simulink model "ofdm_txrx_mimo_2x"  (Simulink 6.6)
settings: Solver=VariableStepDiscrete  StartTime=0.0  StopTime=20e3  RelTol=1e-3
PreLoadFcn:
  | ofdm_rx_mimo_init
InitFcn:
  | ofdm_rx_mimo_init
StartFcn:
  | ofdm_rx_mimo_init
values from neighbouring files (symbols this model uses):
  BER_RAM_init_size = 2048    [ofdm_tx_mimo_init.m]
  CPLength = 16    [ofdm_tx_mimo_init.m]
  CRCPolynomial = hex2dec('04c11db7')    [ofdm_tx_mimo_init.m]
  freqOffset_track_filtCoef_i = hex2dec('2500')/2^32    [ofdm_rx_mimo_init.m]
  freqOffset_track_filtCoef_p = hex2dec('d0000')/2^32    [ofdm_rx_mimo_init.m]
  hi = zeros(1,length(cci))    [ofdm_rx_mimo_init.m]
  hr = zeros(1,length(ccr))    [ofdm_rx_mimo_init.m]
  max_CP_length = 16    [ofdm_tx_mimo_init.m]
  max_num_baseRateSymbols = 31    [ofdm_tx_mimo_init.m]
  max_num_subcarriers = 64    [ofdm_tx_mimo_init.m]
  max_num_trainingSymbols = 15    [ofdm_tx_mimo_init.m]
  max_numBytes = 16384    [ofdm_tx_mimo_init.m]
  max_OFDM_symbols = 2047    [ofdm_tx_mimo_init.m]
  modConstellation_qam16 = .75*[1 3 -1 -3]./3    [ofdm_tx_mimo_init.m]
  modConstellation_qam256 = 0.9375*[3 1 5 7 11 9 13 15 -3 -1 -5 -7 -11 -9 -13 -15]./15    [ofdm_tx_mimo_init.m]
  modConstellation_qam64 = 0.875*[3 1 5 7 -3 -1 -5 -7]./7    [ofdm_tx_mimo_init.m]
  modConstellation_qpsk = [1 -1]./sqrt(2)    [ofdm_tx_mimo_init.m]
  numBaseRateSymbols = 2    [ofdm_tx_mimo_init.m]
  numSubcarriers = 64    [ofdm_tx_mimo_init.m]
  numTrainingSymbols = 2    [ofdm_tx_mimo_init.m]
  pilot1_indicies = 7 + ( (64-7) * 2^16)    (= 3735559)    [ofdm_tx_mimo_init.m]
  pilot2_indicies = 21 + ( (64-21) * 2^16)    (= 2818069)    [ofdm_tx_mimo_init.m]
  pilotValue_neg = hex2dec('8000') + (2^16 * 0)    [ofdm_tx_mimo_init.m]
  pilotValue_pos = hex2dec('7FFF') + (2^16 * 0)    [ofdm_tx_mimo_init.m]
  pkt_crossCorr_thresh = 0.7    [ofdm_rx_mimo_init.m]
  pkt_energy_thresh = 0    [ofdm_rx_mimo_init.m]
  pkt_numPayloadBytes = 1400+42-6    (= 1436)    [ofdm_tx_mimo_init.m]
  pktByteNums = numHeaderBytes +  				byteIndex_numPayloadBytes(1)*2^8 +  				byteIndex_numPayloadBytes(2)*2^16 +  				byteIndex_simpleDynModMasks * 2^24    [ofdm_rx_mimo_init.m]
  PktDet_Delay = 26 + Symbol_Timing_Offset*2^7    (= 1050)    [ofdm_rx_mimo_init.m]
  preamble = 6*[0 shortsyms_10 longsyms_2]    [ofdm_tx_mimo_init.m]
  preamble_I = real(preamble)    [ofdm_tx_mimo_init.m]
  preamble_Q = imag(preamble)    [ofdm_tx_mimo_init.m]
  RAM_init_size = max_numBytes    (= 16384)    [ofdm_tx_mimo_init.m]
  reg_Interrupt_PktBuf_Control = reg_InterruptControl +  	1 * 2^16 +  	0 * 2^24    (= 65656)    [ofdm_rx_mimo_init.m]
  rx_controlBits = 1 * 2  		 + 1 * 4  		 + 0 * 8  		 + 1 * 16 * rx_SISO_Mode  		 + 1 * 32  		 + 1 * 64  		 + 0 * 128  		 + 1 * 256  		 + 0 * 512  		 + 1 * 1024  		 + 0 * 2048  		 + 1 * 4096  		 + 1 * 8192  		 + 0 * 16384  		 + 1 * 65536  		 + 1 * 131072  		 + 0 * 262144  		 + 1 * 524288    [ofdm_rx_mimo_init.m]
  Rx_PhaseNoiseTrack_K = 0.6094    [ofdm_rx_mimo_init.m]
  rx_postEq_scaling = round(rxScaling*2^11) * (1 + 2^16)    [ofdm_rx_mimo_init.m]
  RxDataScrambling_Seq = [40 198 78 63 82 173 102 245 48 111 172 115 147 230 216 93 72 65 62 2 205 242 122 90 128 83 105 97 73 10 5 252]    [ofdm_tx_mimo_init.m]
  simOnly_numSamples = length(preamble)+( (numSubcarriers+CPLength)*(numTrainingSymbols + numBaseRateSymbols + numFullRateSymbols/2) )    [ofdm_tx_mimo_init.m]
  train = [train train]    [ofdm_tx_mimo_init.m]
  tx_controlBits = (antB_preambleShift * 2^4) + (2^0 * tx_SISO_Mode) + 4*0 + 8*1    (= 73)    [ofdm_tx_mimo_init.m]
  TxDataScrambling_Seq = [40 198 78 63 82 173 102 245 48 111 172 115 147 230 216 93 72 65 62 2 205 242 122 90 128 83 105 97 73 10 5 252]    [ofdm_tx_mimo_init.m]
  txReg_symbolCounts = (2^16 * numFullRateSymbols) + (2^8 *numBaseRateSymbols ) + numTrainingSymbols    [ofdm_tx_mimo_init.m]
  TxRx_FFTScaling = bin2dec('010111') + (bin2dec('000101') * 2^6)    [ofdm_tx_mimo_init.m]
undefined symbols (not defined in the file or in its folder): AntA, AntB, BaseRate, CRC_table_gen, gcbh, intfilt, pktTiming_count_payloadStart, pktTiming_symbol_length, xlBlockLoadCallback, xlGetSimulinkPeriod
libraries: xbsIndex_r4 x3973, dspsigops x4, commchan3 x2, commrflib2 x2, dspsrcs4 x1
(part 5 of 38)

== system ofdm_txrx_mimo_2x/OFDM Rx MIMO/Packet_Detection ==
  note: Only use one antenna for packet detection in this version
  note: Delay to match latencies of correlation &
      | energy calculation branches
  note: This block is used to detect the start of a packet. It correlates the energy in the received signal in order to
      | determine the packet start.  The signal will be detected in the preamble  (Usually during the third short symbol).
  note: Pkt Det goes high sometime
      | during the third short symbol
  note: Latency = 2
  note: Latency = 3
  note: Latency = 4
  note: Latency = 2
  note: Latency = 1
  AntA I : Inport
  AntA Q : Inport  Port=2
  AntB I : Inport  Port=3
  AntB Q : Inport  Port=4
  A*conj(B) 4xCLK : Subsystem
  Coarse Pkt Det : Scope  NumInputPorts=6
      settings: YMin=-0.03~-1~-1~-1~-1~-5  YMax=0.04~1~1~1~1~5  SaveName=ScopeData28
  Constant2 : Constant  Value=0
  Convert : xbsIndex_r4/Convert  arith_type="Signed  (2's comp)"  n_bits=18  bin_pt=11  quantization=Truncate  overflow=Flag as error  latency=0  dbl_ovrd=off  pipeline=off  block_type=convert
      settings: xl_area=[0 0 0 0 0 0 0]  block_version=VER_STRING_GOES_HERE
  Delay : xbsIndex_r4/Delay  en=off  latency=16  dbl_ovrd=off  reg_retiming=off  block_type=delay
      settings: infoedit=Hardware notes: A delay line is a chain, each link of which is an SRL16 followed by a flip-flop. If register retiming is enabled, the delay line is a chain of flip-flops.  xl_area=[7 14 0 14 0 0 0]  block_version=VER_STRING_GOES_HERE
  Delay1 : xbsIndex_r4/Delay  en=off  latency=16  dbl_ovrd=off  reg_retiming=off  block_type=delay
      settings: infoedit=Hardware notes: A delay line is a chain, each link of which is an SRL16 followed by a flip-flop. If register retiming is enabled, the delay line is a chain of flip-flops.  xl_area=[7 14 0 14 0 0 0]  block_version=VER_STRING_GOES_HERE
  Delay2 : xbsIndex_r4/Delay  Orientation=down  en=off  latency=3  dbl_ovrd=off  reg_retiming=off  block_type=delay
      settings: infoedit=Hardware notes: A delay line is a chain, each link of which is an SRL16 followed by a flip-flop. If register retiming is enabled, the delay line is a chain of flip-flops.  xl_area=[7 14 0 14 0 0 0]  block_version=VER_STRING_GOES_HERE
  Divide : Product  Inputs=*/  OutScaling=2^-10
  Down Sample : xbsIndex_r4/Down Sample  sample_ratio=2  sample_phase="Last Value of Frame  (most efficient)"  en=off  latency=1  dbl_ovrd=off  block_type=dsamp
      settings: block_version=VER_STRING_GOES_HERE
  Inverter1 : xbsIndex_r4/Inverter  en=off  latency=0  dbl_ovrd=off  block_type=inv
      settings: xl_area=[0 0 0 0 0 0 0]  block_version=VER_STRING_GOES_HERE
  Logical{,1..2,4} (4 alike) : xbsIndex_r4/Logical  logical_function=AND  inputs=2  en=off  latency=0  precision=Full  arith_type=Unsigned  n_bits=8  bin_pt=2  align_bp=on  dbl_ovrd=off  block_type=logical
      settings: xl_area=[1 0 0 1 0 0 0]  block_version=VER_STRING_GOES_HERE
  Logical3 : xbsIndex_r4/Logical  logical_function=OR  inputs=2  en=off  latency=0  precision=Full  arith_type=Unsigned  n_bits=8  bin_pt=2  align_bp=on  dbl_ovrd=off  block_type=logical
      settings: xl_area=[1 0 0 1 0 0 0]  block_version=VER_STRING_GOES_HERE
  Magnitude Squared : Subsystem  mask{prec=22, bp=14, multLatency=3, addLatency=1}
      mask prompts: prec "Precision"; bp "Binary Point"; multLatency "Multiplier Latency"; addLatency "Adder Latency"
  Magnitude Squared1 : Subsystem  mask{prec=17, bp=14, multLatency=2, addLatency=0}
      mask prompts: prec "Precision"; bp "Binary Point"; multLatency "Multiplier Latency"; addLatency "Adder Latency"
  Mult1 : xbsIndex_r4/Mult  precision=User Defined  arith_type=Unsigned  n_bits=18  bin_pt=10  quantization=Truncate  overflow=Flag as error  en=off  latency=2  dbl_ovrd=off  use_behavioral_HDL=off  use_embedded=on  opt=Speed  optimum_pipeline=off  pipeline=on  use_rpm=off  placement_style=Rectangular shape  block_type=mult
      settings: infoedit=Hardware notes: To use the internal pipeline stage of the dedicated multiplier you must select 'Pipeline for maximum performance'.  xl_area=[22 24 0 44 0 1 0]  block_version=VER_STRING_GOES_HERE
  Mult2 : xbsIndex_r4/Mult  precision=User Defined  arith_type=Unsigned  n_bits=22  bin_pt=14  quantization=Truncate  overflow=Flag as error  en=off  latency=2  dbl_ovrd=off  use_behavioral_HDL=off  use_embedded=on  opt=Speed  optimum_pipeline=off  pipeline=on  use_rpm=off  placement_style=Rectangular shape  block_type=mult
      settings: infoedit=Hardware notes: To use the internal pipeline stage of the dedicated multiplier you must select 'Pipeline for maximum performance'.  xl_area=[19 30 0 38 0 2 0]  block_version=VER_STRING_GOES_HERE
  Relational : xbsIndex_r4/Relational  mode=a>b  en=off  latency=1  dbl_ovrd=off  block_type=relational
      settings: xl_area=[11 1 0 22 0 0 0]  block_version=VER_STRING_GOES_HERE
  Relational1 : xbsIndex_r4/Relational  mode=a>b  en=off  latency=3  dbl_ovrd=off  block_type=relational
      settings: xl_area=[10 2 0 19 0 0 0]  block_version=VER_STRING_GOES_HERE
  Rx_ExtPktDet : xbsIndex_r4/Gateway In  arith_type=Boolean  n_bits=1  bin_pt=0  quantization="Round  (unbiased: +/- Inf)"  overflow=Saturate  period=4  dbl_ovrd=off  timing_constraint=None  locs_specified=off  LOCs={}  block_type=gatewayin
      settings: infoedit="Gateway in block.  Converts inputs of type Simulink integer, double and fixed point to  Xilinx fixed point type.<P><P>Hardware notes:  In hardware these blocks become top level input ports."  block_version=VER_STRING_GOES_HERE
  Slice1 : xbsIndex_r4/Slice  nbits=12  boolean_output=off  mode=Lower Bit Location + Width  bit1=0  base1=MSB of Input  bit0=8  base0=LSB of Input  dbl_ovrd=off  block_type=slice
      settings: infoedit="Extracts a given range of bits from each input sample and presents it at the output.  The output type is ordinarily unsigned with binary point at zero, but can be Boolean when the slice is one bit wide.<P><P>Hardware notes: In hardware this block costs nothing."  block_version=VER_STRING_GOES_HERE
  Slice25 : xbsIndex_r4/Slice  nbits=8  boolean_output=off  mode=Lower Bit Location + Width  bit1=0  base1=MSB of Input  bit0=0  base0=LSB of Input  dbl_ovrd=off  block_type=slice
      settings: infoedit="Extracts a given range of bits from each input sample and presents it at the output.  The output type is ordinarily unsigned with binary point at zero, but can be Boolean when the slice is one bit wide.<P><P>Hardware notes: In hardware this block costs nothing."  block_version=VER_STRING_GOES_HERE
  Sliding Window : Subsystem
  Sliding Window1 : Subsystem
  Sliding Window2 : Subsystem
  Terminator : Terminator
  Terminator1 : Terminator
  To_UFix12_5 : xbsIndex_r4/Reinterpret  force_arith_type=on  arith_type=Unsigned  force_bin_pt=on  bin_pt=5  block_type=cast
      settings: infoedit="Changes type of samples without altering their binary representation.<P><P>Hardware notes: In hardware this block costs nothing.<P><P>Example:  Suppose the input is 6 bits wide, signed, with 2 fractional bits, and the output is forced to unsigned with 0 fractional bits.  Then an input of -2.0 (1110.00 in binary 2's complement) becomes an output of 56 (111000 in binary)."  block_version=VER_STRING_GOES_HERE
  To_UFix8_7 : xbsIndex_r4/Reinterpret  force_arith_type=on  arith_type=Unsigned  force_bin_pt=on  bin_pt=7  block_type=cast
      settings: infoedit="Changes type of samples without altering their binary representation.<P><P>Hardware notes: In hardware this block costs nothing.<P><P>Example:  Suppose the input is 6 bits wide, signed, with 2 fractional bits, and the output is forced to unsigned with 0 fractional bits.  Then an input of -2.0 (1110.00 in binary 2's complement) becomes an output of 56 (111000 in binary)."  block_version=VER_STRING_GOES_HERE
  done{1,3..6} (5 alike) : xbsIndex_r4/Gateway Out  hdl_port=off  timing_constraint=None  locs_specified=off  LOCs={}  block_type=gatewayout
      settings: infoedit="Gateway out block.  Converts Xilinx fixed point inputs into ouputs of type Simulink integer, double, or fixed point.<P><P>Hardware notes:  In hardware these blocks become top level output ports or are discarded, depending on how they are configured."  xl_area=[0 0 0 0 1 0 0]  block_version=VER_STRING_GOES_HERE
  Pkt Det : Outport
  wires:
  Constant2/1 -> Rx_ExtPktDet/1
  Logical4/1 -> Logical3/2
  [regRx_enIntPktDet] -> Logical4/1
  [regRx_enExtPktDet] -> Logical2/2
  Logical3/1 -> Pkt Det/1
  Logical2/1 -> Logical3/1
  Rx_ExtPktDet/1 -> Logical2/1
  Divide/1 -> Coarse Pkt Det/6
  done6/1 -> Coarse Pkt Det/4
  done3/1 -> Divide/2, Coarse Pkt Det/3
  done5/1 -> Divide/1, Coarse Pkt Det/2
  done4/1 -> Coarse Pkt Det/1
  done1/1 -> Coarse Pkt Det/5
  Down Sample/1 -> Inverter1/1
  AntB Q/1 -> Terminator1/1
  AntB I/1 -> Terminator/1
  Delay2/1 -> done5/1, Relational/1
  Logical/1 -> done6/1, Logical1/1
  Logical1/1 -> Logical4/2, done1/1
  Inverter1/1 -> Logical1/2
  [rx_global_reset] -> Down Sample/1
  Relational1/1 -> Logical/2
  Convert/1 -> Mult1/2, Mult1/1
  Relational/1 -> Logical/1
  Magnitude Squared/1(Mag) -> Delay2/1
  Mult2/1 -> Relational/2
  Mult1/1 -> done3/1, Relational1/1, Mult2/1
  Sliding Window2/1(Out) -> Convert/1
  Magnitude Squared1/1(Mag) -> Sliding Window2/1(In)
  Sliding Window1/1(Out) -> Magnitude Squared/2(Q)
  Sliding Window/1(Out) -> Magnitude Squared/1(I)
  A*conj(B) 4xCLK/2(Im1) -> Sliding Window1/1(In)
  A*conj(B) 4xCLK/1(Re) -> Sliding Window/1(In)
  Delay1/1 -> Magnitude Squared1/2(Q), A*conj(B) 4xCLK/4(Im[B])
  Delay/1 -> Magnitude Squared1/1(I), A*conj(B) 4xCLK/2(Re[B])
  AntA I/1 -> done4/1, A*conj(B) 4xCLK/1(Re[A]), Delay/1
  AntA Q/1 -> A*conj(B) 4xCLK/3(Im[A]), Delay1/1
  Slice1/1 -> To_UFix12_5/1
  [Rx_IntPktDet_Thresholds] -> Slice25/1, Slice1/1
  Slice25/1 -> To_UFix8_7/1
  To_UFix8_7/1 -> Mult2/2
  To_UFix12_5/1 -> Relational1/2

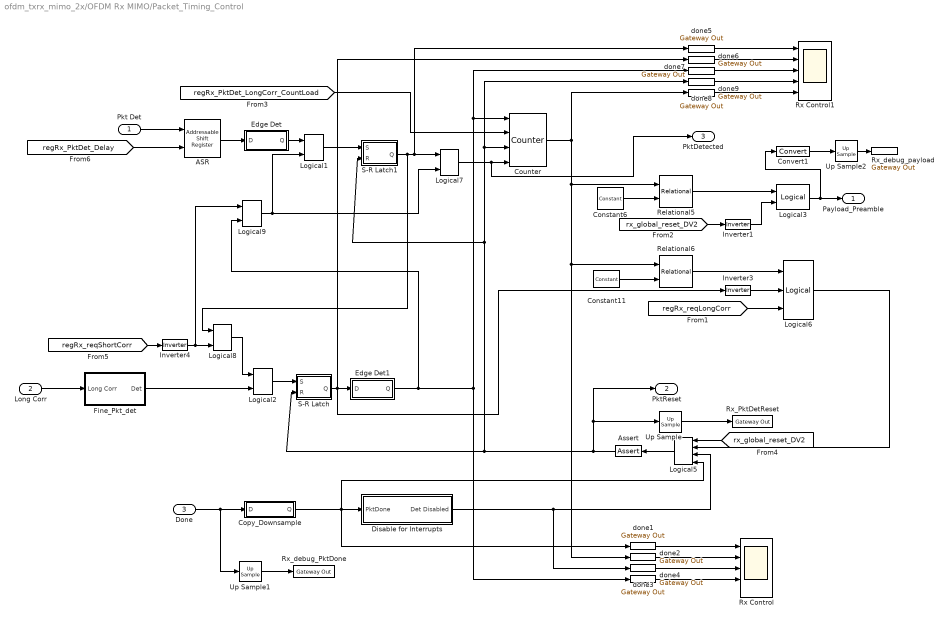
== system ofdm_txrx_mimo_2x/OFDM Rx MIMO/Packet_Timing_Control ==
  Pkt Det : Inport
  Long Corr : Inport  Port=2
  Done : Inport  Port=3
  ASR : xbsIndex_r4/Addressable Shift Register  infer_latency=on  depth=2  initVector=[0]  en=off  dbl_ovrd=off  use_behavioral_HDL=off  use_rpm=off  block_type=addrsr
      settings: infoedit="Delay of  configurable length.  Any element  in the delay     line   can    be   addressed   and   driven    onto   the   output     port.<P><P>Hardware  notes: Implemented  using SRL16s.     If Virtex-4, Virtex-II or Spartan-3 devices are used, multiple SRLC16s are cascaded together."  xl_area=[13 0 0 25 0 0 0]  block_version=VER_STRING_GOES_HERE
  Assert : xbsIndex_r4/Assert  Orientation=left  assert_type=on  type_source=Explicitly  arith_type=Boolean  n_bits=16  bin_pt=14  assert_rate=on  rate_source=Explicitly  period=4  output_port=on  block_type=assert
      settings: infoedit=Asserts a user-defined sample rate and/or type on a signal.<P><P>Hardware notes: In hardware this block costs nothing.  block_version=VER_STRING_GOES_HERE
  Constant11 : xbsIndex_r4/Constant  arith_type=Unsigned  const=pktTiming_count_payloadStart-2  n_bits=ceil(log2(pktTiming_count_payloadStart))  bin_pt=0  explicit_period=off  period=1  equ=P=C  opselect=C  inp2=PCIN>>17  opr=+  inp1=P  carry=CIN  dbl_ovrd=off  block_type=constant
      settings: block_version=VER_STRING_GOES_HERE
  Constant6 : xbsIndex_r4/Constant  arith_type=Unsigned  const=pktTiming_count_payloadStart  n_bits=ceil(log2(pktTiming_count_payloadStart))+1  bin_pt=0  explicit_period=off  period=1  equ=P=C  opselect=C  inp2=PCIN>>17  opr=+  inp1=P  carry=CIN  dbl_ovrd=off  block_type=constant
      settings: block_version=VER_STRING_GOES_HERE
  Convert1 : xbsIndex_r4/Convert  arith_type=Unsigned  n_bits=1  bin_pt=0  quantization=Truncate  overflow=Wrap  latency=0  dbl_ovrd=off  pipeline=off  block_type=convert
      settings: xl_area=[0, 0, 0, 0, 0, 0, 0]  block_version=VER_STRING_GOES_HERE
  Copy_Downsample : Subsystem
  Counter : xbsIndex_r4/Counter  cnt_type=Free Running  cnt_to=Inf  operation=Up  start_count=0  cnt_by_val=1  arith_type=Unsigned  n_bits=pktTiming_controlCounter_bits  bin_pt=0  load_pin=on  rst=on  en=on  explicit_period=off  period=1  dbl_ovrd=off  use_behavioral_HDL=off  use_rpm=on  implementation=Fabric  block_type=counter
      settings: xl_area=[6 11 0 12 0 0 0]  block_version=VER_STRING_GOES_HERE
  Disable for Interrupts : Subsystem
  Edge Det : Subsystem
  Edge Det1 : Subsystem
  Fine_Pkt_det : Subsystem
  Inverter1 : xbsIndex_r4/Inverter  en=off  latency=0  dbl_ovrd=off  block_type=inv
      settings: xl_area=[0 0 0 0 0 0 0]  block_version=VER_STRING_GOES_HERE
  Inverter3 : xbsIndex_r4/Inverter  en=off  latency=0  dbl_ovrd=off  block_type=inv
      settings: xl_area=[0 0 0 0 0 0 0]  block_version=VER_STRING_GOES_HERE
  Inverter4 : xbsIndex_r4/Inverter  en=off  latency=0  dbl_ovrd=off  block_type=inv
      settings: xl_area=[0 0 0 0 0 0 0]  block_version=VER_STRING_GOES_HERE
  Logical1 : xbsIndex_r4/Logical  logical_function=OR  inputs=2  en=off  latency=0  precision=Full  arith_type=Unsigned  n_bits=8  bin_pt=2  align_bp=on  dbl_ovrd=off  block_type=logical
      settings: xl_area=[1 0 0 1 0 0 0]  block_version=VER_STRING_GOES_HERE
  Logical2 : xbsIndex_r4/Logical  logical_function=AND  inputs=2  en=off  latency=0  precision=Full  arith_type=Unsigned  n_bits=8  bin_pt=2  align_bp=on  dbl_ovrd=off  block_type=logical
      settings: xl_area=[1 0 0 1 0 0 0]  block_version=VER_STRING_GOES_HERE
  Logical3 : xbsIndex_r4/Logical  logical_function=AND  inputs=2  en=off  latency=0  precision=Full  arith_type=Unsigned  n_bits=8  bin_pt=2  align_bp=on  dbl_ovrd=off  block_type=logical
      settings: xl_area=[1 0 0 1 0 0 0]  block_version=VER_STRING_GOES_HERE
  Logical5 : xbsIndex_r4/Logical  Orientation=left  logical_function=OR  inputs=4  en=off  latency=0  precision=Full  arith_type=Unsigned  n_bits=8  bin_pt=2  align_bp=on  dbl_ovrd=off  block_type=logical
      settings: xl_area=[1 0 0 1 0 0 0]  block_version=VER_STRING_GOES_HERE
  Logical6 : xbsIndex_r4/Logical  logical_function=AND  inputs=3  en=off  latency=0  precision=Full  arith_type=Unsigned  n_bits=8  bin_pt=2  align_bp=on  dbl_ovrd=off  block_type=logical
      settings: xl_area=[1 0 0 1 0 0 0]  block_version=VER_STRING_GOES_HERE
  Logical7 : xbsIndex_r4/Logical  logical_function=OR  inputs=2  en=off  latency=0  precision=Full  arith_type=Unsigned  n_bits=8  bin_pt=2  align_bp=on  dbl_ovrd=off  block_type=logical
      settings: xl_area=[1 0 0 1 0 0 0]  block_version=VER_STRING_GOES_HERE
  Logical8 : xbsIndex_r4/Logical  logical_function=OR  inputs=2  en=off  latency=0  precision=Full  arith_type=Unsigned  n_bits=8  bin_pt=2  align_bp=on  dbl_ovrd=off  block_type=logical
      settings: xl_area=[1 0 0 1 0 0 0]  block_version=VER_STRING_GOES_HERE
  Logical9 : xbsIndex_r4/Logical  logical_function=AND  inputs=2  en=off  latency=0  precision=Full  arith_type=Unsigned  n_bits=8  bin_pt=2  align_bp=on  dbl_ovrd=off  block_type=logical
      settings: xl_area=[1 0 0 1 0 0 0]  block_version=VER_STRING_GOES_HERE
  Relational5 : xbsIndex_r4/Relational  mode=a>b  en=off  latency=0  dbl_ovrd=off  block_type=relational
      settings: xl_area=[6 0 0 11 0 0 0]  block_version=VER_STRING_GOES_HERE
  Relational6 : xbsIndex_r4/Relational  mode=a>b  en=off  latency=0  dbl_ovrd=off  block_type=relational
      settings: xl_area=[6 0 0 11 0 0 0]  block_version=VER_STRING_GOES_HERE
  Rx Control : Scope  NumInputPorts=5
      settings: TimeRange=2500  YMin=0.95~885~-1~-1~-1  YMax=1.05~935~1~1~1  SaveName=ScopeData13
  Rx Control1 : Scope  NumInputPorts=5
      settings: TimeRange=2500  YMin=1~1~-1~-1~605  YMax=1~1~1~1~665  SaveName=ScopeData47
  Rx_PktDetReset : xbsIndex_r4/Gateway Out  hdl_port=on  timing_constraint=None  locs_specified=off  LOCs={}  block_type=gatewayout
      settings: infoedit="Gateway out block.  Converts Xilinx fixed point inputs into ouputs of type Simulink integer, double, or fixed point.<P><P>Hardware notes:  In hardware these blocks become top level output ports or are discarded, depending on how they are configured."  xl_area=[0, 0, 0, 0, 0, 0, 0]  block_version=VER_STRING_GOES_HERE
  Rx_debug_PktDone : xbsIndex_r4/Gateway Out  hdl_port=on  timing_constraint=None  locs_specified=off  LOCs={}  block_type=gatewayout
      settings: infoedit="Gateway out block.  Converts Xilinx fixed point inputs into ouputs of type Simulink integer, double, or fixed point.<P><P>Hardware notes:  In hardware these blocks become top level output ports or are discarded, depending on how they are configured."  xl_area=[0, 0, 0, 0, 0, 0, 0]  block_version=VER_STRING_GOES_HERE
  Rx_debug_payload : xbsIndex_r4/Gateway Out  hdl_port=on  timing_constraint=None  locs_specified=off  LOCs={}  block_type=gatewayout
      settings: infoedit="Gateway out block.  Converts Xilinx fixed point inputs into ouputs of type Simulink integer, double, or fixed point.<P><P>Hardware notes:  In hardware these blocks become top level output ports or are discarded, depending on how they are configured."  xl_area=[0 0 0 0 1 0 0]  block_version=VER_STRING_GOES_HERE
  S-R Latch : Subsystem
  S-R Latch1 : Subsystem
  Up Sample : xbsIndex_r4/Up Sample  sample_ratio=4  copy_samples=on  dbl_ovrd=off  block_type=usamp
      settings: infoedit="Up samples input data.  Inserted values  can be zeros or copies of the most recent input sample.<P><P>Hardware notes: No hardware is needed if inserted values are copies of the input sample; otherwise, a mux and single bit flip-flop are used."  block_version=8.2.02
  Up Sample1 : xbsIndex_r4/Up Sample  sample_ratio=2  copy_samples=on  dbl_ovrd=off  block_type=usamp
      settings: infoedit="Up samples input data.  Inserted values  can be zeros or copies of the most recent input sample.<P><P>Hardware notes: No hardware is needed if inserted values are copies of the input sample; otherwise, a mux and single bit flip-flop are used."  block_version=8.2.02
  Up Sample2 : xbsIndex_r4/Up Sample  sample_ratio=4  copy_samples=on  dbl_ovrd=off  block_type=usamp
      settings: infoedit="Up samples input data.  Inserted values  can be zeros or copies of the most recent input sample.<P><P>Hardware notes: No hardware is needed if inserted values are copies of the input sample; otherwise, a mux and single bit flip-flop are used."  block_version=8.2.02
  done{1..9} (9 alike) : xbsIndex_r4/Gateway Out  hdl_port=off  timing_constraint=None  locs_specified=off  LOCs={}  block_type=gatewayout
      settings: infoedit="Gateway out block.  Converts Xilinx fixed point inputs into ouputs of type Simulink integer, double, or fixed point.<P><P>Hardware notes:  In hardware these blocks become top level output ports or are discarded, depending on how they are configured."  xl_area=[0 0 0 0 1 0 0]  block_version=VER_STRING_GOES_HERE
  Payload_Preamble : Outport
  PktReset : Outport  Port=2
  PktDetected : Outport  Port=3
  wires:
  Convert1/1 -> Up Sample2/1
  done9/1 -> Rx Control1/5
  done8/1 -> Rx Control1/4
  done7/1 -> Rx Control1/3
  done6/1 -> Rx Control1/2
  done5/1 -> Rx Control1/1
  Copy_Downsample/1(Q) -> done1/1, Logical5/4, Disable for Interrupts/1(PktDone)
  Assert/1 -> S-R Latch1/2(R), done8/1, Counter/3, S-R Latch/2(R), PktReset/1, Up Sample/1
  Disable for Interrupts/1(Det Disabled) -> done3/1, Logical5/3
  Done/1 -> Copy_Downsample/1(D), Up Sample1/1
  S-R Latch1/1(Q) -> done5/1, Logical7/1, Logical8/1
  Logical1/1 -> S-R Latch1/1(S)
  S-R Latch/1(Q) -> done6/1, Inverter3/1, Edge Det1/1(D)
  Edge Det1/1(Q) -> done7/1, Counter/1, done4/1, Logical9/2
  Logical9/1 -> Logical7/2, Logical1/2
  [regRx_PktDet_LongCorr_CountLoad] -> Counter/2
  Logical8/1 -> Logical2/1
  Inverter4/1 -> Logical9/1, Logical8/2
  [regRx_reqShortCorr] -> Inverter4/1
  Logical2/1 -> S-R Latch/1(S)
  Long Corr/1 -> Fine_Pkt_det/1(Long Corr)
  done4/1 -> Rx Control/4
  done3/1 -> Rx Control/3
  done2/1 -> Rx Control/2
  done1/1 -> Rx Control/1
  Logical7/1 -> Counter/4, PktDetected/1
  Logical6/1 -> Logical5/2
  [regRx_reqLongCorr] -> Logical6/3
  Inverter3/1 -> Logical6/2
  Relational6/1 -> Logical6/1
  Constant11/1 -> Relational6/2
  [rx_global_reset_DV2] -> Logical5/1
  Logical5/1 -> Assert/1
  Logical3/1 -> Convert1/1, Payload_Preamble/1
  Relational5/1 -> Logical3/1
  Inverter1/1 -> Logical3/2
  [rx_global_reset_DV2] -> Inverter1/1
  [regRx_PktDet_Delay] -> ASR/2
  ASR/1 -> Edge Det/1(D)
  Pkt Det/1 -> ASR/1
  Constant6/1 -> Relational5/2
  Edge Det/1(Q) -> Logical1/1
  Counter/1 -> done2/1, Relational6/1, Relational5/1, done9/1
  Fine_Pkt_det/1(Det) -> Logical2/2
  Up Sample/1 -> Rx_PktDetReset/1
  Up Sample1/1 -> Rx_debug_PktDone/1
  Up Sample2/1 -> Rx_debug_payload/1

== system ofdm_txrx_mimo_2x/OFDM Rx MIMO/Rx Reset Logic ==
  Convert : xbsIndex_r4/Convert  arith_type=Boolean  n_bits=8  bin_pt=6  quantization=Truncate  overflow=Wrap  latency=0  dbl_ovrd=off  pipeline=off  block_type=convert
      settings: xl_area=[0, 0, 0, 0, 0, 0, 0]  block_version=VER_STRING_GOES_HERE
  Down Sample6 : xbsIndex_r4/Down Sample  sample_ratio=2  sample_phase="Last Value of Frame  (most efficient)"  en=off  latency=1  dbl_ovrd=off  block_type=dsamp
      settings: block_version=VER_STRING_GOES_HERE
  Logical1 : xbsIndex_r4/Logical  logical_function=OR  inputs=2  en=off  latency=0  precision=Full  arith_type=Unsigned  n_bits=8  bin_pt=2  align_bp=on  dbl_ovrd=off  block_type=logical
      settings: xl_area=[1 0 0 1 0 0 0]  block_version=VER_STRING_GOES_HERE
  RXreset : Constant  Value=0
  Rx_Reset : xbsIndex_r4/Gateway In  arith_type=Boolean  n_bits=6  bin_pt=0  quantization="Round  (unbiased: +/- Inf)"  overflow=Saturate  period=4  dbl_ovrd=off  timing_constraint=None  locs_specified=off  LOCs={}  block_type=gatewayin
      settings: infoedit="Gateway in block.  Converts inputs of type Simulink integer, double and fixed point to  Xilinx fixed point type.<P><P>Hardware notes:  In hardware these blocks become top level input ports."  xl_area=[0 0 0 0 1 0 0]  block_version=VER_STRING_GOES_HERE
  tag scope: rx_global_reset=global, rx_global_reset_DV2=global
  wires:
  RXreset/1 -> Rx_Reset/1
  [regRx_GlobalReset] -> Logical1/1
  Logical1/1 -> [rx_global_reset], Down Sample6/1
  Rx_Reset/1 -> Convert/1
  Convert/1 -> Logical1/2
  Down Sample6/1 -> [rx_global_reset_DV2]

== system ofdm_txrx_mimo_2x/OFDM Tx MIMO/Filter & DACs ==
  A_I : Inport
  A_Q : Inport  Port=2
  B_I : Inport  Port=3
  B_Q : Inport  Port=4
  Convert : xbsIndex_r4/Convert  arith_type=Boolean  n_bits=16  bin_pt=14  quantization=Truncate  overflow=Wrap  latency=0  dbl_ovrd=off  pipeline=off  block_type=convert
      settings: block_version=8.2.02
  Down Sample : xbsIndex_r4/Down Sample  sample_ratio=2  sample_phase="Last Value of Frame  (most efficient)"  en=off  latency=1  dbl_ovrd=off  block_type=dsamp
      settings: block_version=10.1
  Logical : xbsIndex_r4/Logical  logical_function=AND  inputs=2  en=off  latency=0  precision=Full  arith_type=Unsigned  n_bits=16  bin_pt=0  align_bp=on  dbl_ovrd=off  block_type=logical
      settings: block_version=8.2.02
  Mux : xbsIndex_r4/Mux  inputs=2  en=off  latency=1  precision=Full  arith_type=Unsigned  n_bits=16  bin_pt=14  quantization=Truncate  overflow=Wrap  dbl_ovrd=off  block_type=mux
      settings: block_version=8.2.02
  Mux1 : xbsIndex_r4/Mux  inputs=2  en=off  latency=1  precision=Full  arith_type=Unsigned  n_bits=16  bin_pt=14  quantization=Truncate  overflow=Wrap  dbl_ovrd=off  block_type=mux
      settings: block_version=8.2.02
  Polyphase 2x Interpolator1 : Subsystem  mask{h=[0 intfilt(2,4,0.8)] .* 0.99, polyphaseBranches=2, data_samplePeriod=4, coeff_prec=14, coeff_bp=13, multLatency=1, mult_prec=18, mult_bp=17, add_prec=16, add_bp=15}
      mask prompts: h "Filter Coefficients"; polyphaseBranches "Interpolation Rate"; data_samplePeriod "Input Sample Period"; coeff_prec "Coefficients - Num Bits"; coeff_bp "Coefficients - Binary Point"; multLatency "Multiplier Latency"; mult_prec "Multiplier - Num Bits"; mult_bp "Multiplier - Binary Point"; add_prec "Final Adder - Num Bits"; add_bp "Final Adder - Binary Point"
      mask help:
      | This block implements a interpolating polyphase FIR filter. In this version,
      | the interpolation rate must be 8, and the filter must be of length 64.
      mask init:
      | subFilterLength = 2;
  Polyphase 2x Interpolator2 : Subsystem  mask{h=[0 intfilt(2,4,0.8)] .* 0.99, polyphaseBranches=2, data_samplePeriod=4, coeff_prec=14, coeff_bp=13, multLatency=1, mult_prec=18, mult_bp=17, add_prec=16, add_bp=15}
      mask prompts: h "Filter Coefficients"; polyphaseBranches "Interpolation Rate"; data_samplePeriod "Input Sample Period"; coeff_prec "Coefficients - Num Bits"; coeff_bp "Coefficients - Binary Point"; multLatency "Multiplier Latency"; mult_prec "Multiplier - Num Bits"; mult_bp "Multiplier - Binary Point"; add_prec "Final Adder - Num Bits"; add_bp "Final Adder - Binary Point"
      mask help:
      | This block implements a interpolating polyphase FIR filter. In this version,
      | the interpolation rate must be 8, and the filter must be of length 64.
      mask init:
      | subFilterLength = 2;
  Polyphase 2x Interpolator3 : Subsystem  mask{h=[0 intfilt(2,4,0.8)] .* 0.99, polyphaseBranches=2, data_samplePeriod=4, coeff_prec=14, coeff_bp=13, multLatency=1, mult_prec=18, mult_bp=17, add_prec=16, add_bp=15}
      mask prompts: h "Filter Coefficients"; polyphaseBranches "Interpolation Rate"; data_samplePeriod "Input Sample Period"; coeff_prec "Coefficients - Num Bits"; coeff_bp "Coefficients - Binary Point"; multLatency "Multiplier Latency"; mult_prec "Multiplier - Num Bits"; mult_bp "Multiplier - Binary Point"; add_prec "Final Adder - Num Bits"; add_bp "Final Adder - Binary Point"
      mask help:
      | This block implements a interpolating polyphase FIR filter. In this version,
      | the interpolation rate must be 8, and the filter must be of length 64.
      mask init:
      | subFilterLength = 2;
  Polyphase 2x Interpolator4 : Subsystem  mask{h=[0 intfilt(2,4,0.8)] .* 0.99, polyphaseBranches=2, data_samplePeriod=4, coeff_prec=14, coeff_bp=13, multLatency=1, mult_prec=18, mult_bp=17, add_prec=16, add_bp=15}
      mask prompts: h "Filter Coefficients"; polyphaseBranches "Interpolation Rate"; data_samplePeriod "Input Sample Period"; coeff_prec "Coefficients - Num Bits"; coeff_bp "Coefficients - Binary Point"; multLatency "Multiplier Latency"; mult_prec "Multiplier - Num Bits"; mult_bp "Multiplier - Binary Point"; add_prec "Final Adder - Num Bits"; add_bp "Final Adder - Binary Point"
      mask help:
      | This block implements a interpolating polyphase FIR filter. In this version,
      | the interpolation rate must be 8, and the filter must be of length 64.
      mask init:
      | subFilterLength = 2;
  Register : xbsIndex_r4/Register  init=0  rst=off  en=off  dbl_ovrd=off  block_type=register
  Register1 : xbsIndex_r4/Register  init=0  rst=off  en=off  dbl_ovrd=off  block_type=register
  To Fix16_{1..3,15} (4 alike) : xbsIndex_r4/Convert  arith_type="Signed  (2's comp)"  n_bits=16  bin_pt=15  quantization=Truncate  overflow=Wrap  latency=0  dbl_ovrd=off  pipeline=off  block_type=convert
      settings: block_version=10.1
  Tx_AntA_DAC_I : xbsIndex_r4/Gateway Out  hdl_port=on  timing_constraint=None  locs_specified=off  LOCs={}  block_type=gatewayout
      settings: infoedit="Gateway out block.  Converts Xilinx fixed point inputs into ouputs of type Simulink integer, double, or fixed point.<P><P>Hardware notes:  In hardware these blocks become top level output ports or are discarded, depending on how they are configured."  block_version=10.1.3
  Tx_AntA_DAC_Q : xbsIndex_r4/Gateway Out  hdl_port=on  timing_constraint=None  locs_specified=off  LOCs={}  block_type=gatewayout
      settings: infoedit="Gateway out block.  Converts Xilinx fixed point inputs into ouputs of type Simulink integer, double, or fixed point.<P><P>Hardware notes:  In hardware these blocks become top level output ports or are discarded, depending on how they are configured."  block_version=10.1.3
  Tx_AntB_DAC_I : xbsIndex_r4/Gateway Out  hdl_port=on  timing_constraint=None  locs_specified=off  LOCs={}  block_type=gatewayout
      settings: infoedit="Gateway out block.  Converts Xilinx fixed point inputs into ouputs of type Simulink integer, double, or fixed point.<P><P>Hardware notes:  In hardware these blocks become top level output ports or are discarded, depending on how they are configured."  block_version=10.1.3
  Tx_AntB_DAC_Q : xbsIndex_r4/Gateway Out  hdl_port=on  timing_constraint=None  locs_specified=off  LOCs={}  block_type=gatewayout
      settings: infoedit="Gateway out block.  Converts Xilinx fixed point inputs into ouputs of type Simulink integer, double, or fixed point.<P><P>Hardware notes:  In hardware these blocks become top level output ports or are discarded, depending on how they are configured."  block_version=10.1.3
  " A_I" : Outport
  " A_Q" : Outport  Port=2
  " B_I" : Outport  Port=3
  " B_Q" : Outport  Port=4
  wires:
  Polyphase 2x Interpolator3/1(Y) -> Mux/3, Register/1
  Tx_AntA_DAC_I/1 -> " A_I"/1
  Polyphase 2x Interpolator1/1(Y) -> Mux1/3, Register1/1
  Tx_AntA_DAC_Q/1 -> " A_Q"/1
  Polyphase 2x Interpolator2/1(Y) -> Mux/2
  Tx_AntB_DAC_I/1 -> " B_I"/1
  Polyphase 2x Interpolator4/1(Y) -> Mux1/2
  Tx_AntB_DAC_Q/1 -> " B_Q"/1
  Mux/1 -> To Fix16_2/1
  Mux1/1 -> To Fix16_3/1
  Logical/1 -> Down Sample/1
  Register/1 -> To Fix16_15/1
  Register1/1 -> To Fix16_1/1
  [TxReg_SISO_Mode] -> Logical/1
  [TxReg_SISO_AntB] -> Convert/1
  Convert/1 -> Logical/2
  Down Sample/1 -> Mux/1, Mux1/1
  To Fix16_15/1 -> Tx_AntA_DAC_I/1
  To Fix16_1/1 -> Tx_AntA_DAC_Q/1
  To Fix16_2/1 -> Tx_AntB_DAC_I/1
  To Fix16_3/1 -> Tx_AntB_DAC_Q/1
  A_I/1 -> Polyphase 2x Interpolator3/1(X)
  A_Q/1 -> Polyphase 2x Interpolator1/1(X)
  B_I/1 -> Polyphase 2x Interpolator2/1(X)
  B_Q/1 -> Polyphase 2x Interpolator4/1(X)

== system ofdm_txrx_mimo_2x/OFDM Tx MIMO/IFFT ==
  xn_I : Inport
  xn_Q : Inport  Port=2
  Start : Inport  Port=3
  Constant3 : xbsIndex_r4/Constant  arith_type=Boolean  const=0  n_bits=1  bin_pt=0  explicit_period=off  period=1  equ=P=C  opselect=C  inp2=PCIN>>17  opr=+  inp1=P  carry=CIN  dbl_ovrd=off  block_type=constant
      settings: block_version=VER_STRING_GOES_HERE
  Constant4 : xbsIndex_r4/Constant  arith_type=Boolean  const=1  n_bits=1  bin_pt=0  explicit_period=off  period=1  equ=P=C  opselect=C  inp2=PCIN>>17  opr=+  inp1=P  carry=CIN  dbl_ovrd=off  block_type=constant
      settings: block_version=VER_STRING_GOES_HERE
  Constant6 : xbsIndex_r4/Constant  arith_type=Boolean  const=1  n_bits=1  bin_pt=0  explicit_period=off  period=1  equ=P=C  opselect=C  inp2=PCIN>>17  opr=+  inp1=P  carry=CIN  dbl_ovrd=off  block_type=constant
      settings: block_version=VER_STRING_GOES_HERE
  FFTx : xbsIndex_r4/FFT v3_1   arch=Pipelined Streaming I/O  npts=64  scaling=Scaled  rounding=Convergent Rounding  tf_width=16  en_n=off  has_ovflo=on  rst=on  en=off  op_order=Natural Order  dbl_ovrd=off  explicit_period=off  period=4  use_dsp48=off  twiddle_mem_type=Block RAM  data_mem_type=Block RAM  num_bram_stages=3  block_type=fftx
      settings: infoedit=Devices Supported: Virtex-4, Virtex-II, Virtex-IIPro, Spartan-3 and Spartan-3E  xl_area=[0, 0, 0, 0, 0, 0, 0]  block_version=VER_STRING_GOES_HERE
  IFFT Input : Scope  NumInputPorts=4
      settings: TimeRange=4000  YMin=-0.8~-0.8~-1~0  YMax=1~0.8~1~65  SaveName=ScopeData16
  IFFT Output : Scope  NumInputPorts=6
      settings: TimeRange=4000  YMin=-0.6~-0.6~0~0~0~-1  YMax=0.2~0.2~70~1~1~1  SaveName=ScopeData17
  IFFT Output1 : Scope  NumInputPorts=6
      settings: TimeRange=4000  YMin=-0.6~-0.6~0~0~0~-1  YMax=0.2~0.2~70~1~1~1  SaveName=ScopeData69
  done{1..10} (10 alike) : xbsIndex_r4/Gateway Out  hdl_port=off  timing_constraint=None  locs_specified=off  LOCs={}  block_type=gatewayout
      settings: infoedit="Gateway out block.  Converts Xilinx fixed point inputs into ouputs of type Simulink integer, double, or fixed point.<P><P>Hardware notes:  In hardware these blocks become top level output ports or are discarded, depending on how they are configured."  xl_area=[0 0 0 0 1 0 0]  block_version=VER_STRING_GOES_HERE
  xn_Index : Outport
  Vout : Outport  Port=2
  xk_I : Outport  Port=3
  xk_Q : Outport  Port=4
  xk_Index : Outport  Port=5
  wires:
  Constant3/1 -> FFTx/4
  Constant4/1 -> FFTx/5
  Constant6/1 -> FFTx/7
  [TxReset] -> FFTx/8
  xn_I/1 -> FFTx/1, done4/1
  FFTx/1 -> xk_I/1, done8/1
  FFTx/2 -> xk_Q/1, done9/1
  xn_Q/1 -> FFTx/2, done5/1
  FFTx/3 -> xn_Index/1, done6/1, IFFT Output1/2
  Start/1 -> FFTx/3, done3/1, IFFT Output1/1
  FFTx/4 -> xk_Index/1, done7/1, IFFT Output1/3
  FFTx/7 -> Vout/1, done10/1, IFFT Output1/4
  done4/1 -> IFFT Input/1
  done5/1 -> IFFT Input/2
  done3/1 -> IFFT Input/3
  done6/1 -> IFFT Input/4
  done8/1 -> IFFT Output/1
  done9/1 -> IFFT Output/2
  done7/1 -> IFFT Output/3
  done10/1 -> IFFT Output/4
  done2/1 -> IFFT Output/5
  FFTx/9 -> done2/1, IFFT Output1/5
  done1/1 -> IFFT Output/6
  FFTx/10 -> done1/1
  [Tx_FFTScaling] -> FFTx/6

== system ofdm_txrx_mimo_2x/OFDM Tx MIMO/OutputBuffers ==
  IFFT Vout : Inport
  I : Inport  Port=2
  Q : Inport  Port=3
  Xk_index : Inport  Port=4
  1 Shift Reg : Subsystem
  Addr Gen : Subsystem
  Constant2 : xbsIndex_r4/Constant  arith_type=Boolean  const=0  n_bits=0  bin_pt=0  explicit_period=off  period=1  equ=P=C  opselect=C  inp2=PCIN>>17  opr=+  inp1=P  carry=CIN  dbl_ovrd=off  block_type=constant
      settings: block_version=VER_STRING_GOES_HERE
  Convert1 : xbsIndex_r4/Convert  arith_type="Signed  (2's comp)"  n_bits=14  bin_pt=13  quantization=Truncate  overflow=Flag as error  latency=0  dbl_ovrd=off  pipeline=off  block_type=convert
      settings: xl_area=[0 0 0 0 0 0 0]  block_version=VER_STRING_GOES_HERE
  Convert2 : xbsIndex_r4/Convert  arith_type="Signed  (2's comp)"  n_bits=14  bin_pt=13  quantization=Truncate  overflow=Flag as error  latency=0  dbl_ovrd=off  pipeline=off  block_type=convert
      settings: xl_area=[0 0 0 0 0 0 0]  block_version=VER_STRING_GOES_HERE
  Delay : xbsIndex_r4/Delay  en=off  latency=1  dbl_ovrd=off  reg_retiming=off  block_type=delay
      settings: infoedit=Hardware notes: A delay line is a chain, each link of which is an SRL16 followed by a flip-flop. If register retiming is enabled, the delay line is a chain of flip-flops.  block_version=VER_STRING_GOES_HERE
  Logical : xbsIndex_r4/Logical  logical_function=AND  inputs=2  en=off  latency=0  precision=Full  arith_type=Unsigned  n_bits=16  bin_pt=0  align_bp=on  dbl_ovrd=off  block_type=logical
      settings: block_version=VER_STRING_GOES_HERE
  Logical1 : xbsIndex_r4/Logical  logical_function=AND  inputs=2  en=off  latency=0  precision=Full  arith_type=Unsigned  n_bits=16  bin_pt=0  align_bp=on  dbl_ovrd=off  block_type=logical
      settings: block_version=VER_STRING_GOES_HERE
  Logical2 : xbsIndex_r4/Logical  logical_function=OR  inputs=2  en=off  latency=0  precision=Full  arith_type=Unsigned  n_bits=16  bin_pt=0  align_bp=on  dbl_ovrd=off  block_type=logical
      settings: block_version=VER_STRING_GOES_HERE
  Logical3 : xbsIndex_r4/Logical  logical_function=AND  inputs=2  en=off  latency=0  precision=Full  arith_type=Unsigned  n_bits=16  bin_pt=0  align_bp=on  dbl_ovrd=off  block_type=logical
      settings: block_version=VER_STRING_GOES_HERE
  Logical4 : xbsIndex_r4/Logical  logical_function=OR  inputs=2  en=off  latency=0  precision=Full  arith_type=Unsigned  n_bits=16  bin_pt=0  align_bp=on  dbl_ovrd=off  block_type=logical
      settings: block_version=VER_STRING_GOES_HERE
  Logical5 : xbsIndex_r4/Logical  logical_function=OR  inputs=2  en=off  latency=0  precision=Full  arith_type=Unsigned  n_bits=16  bin_pt=0  align_bp=on  dbl_ovrd=off  block_type=logical
      settings: block_version=VER_STRING_GOES_HERE
  Mem Control : Subsystem
  Output Buffer Scope : Scope  NumInputPorts=7
      settings: TimeRange=3000  YMin=0~0~0~0~0~0~0  YMax=1~1~1~1~150~150~1  SaveName=ScopeData5
  Pulse Generator1 : DiscretePulseGenerator  Period=simOnly_numSamples*2 + 500  PhaseDelay=(simOnly_numSamples*2 + 500) - 300
  RAMs : Subsystem
  Symbol Counter : xbsIndex_r4/Counter  cnt_type=Free Running  cnt_to=Inf  operation=Up  start_count=0  cnt_by_val=1  arith_type="Signed  (2's comp)"  n_bits=ceil(log2(max_OFDM_symbols+1))  bin_pt=0  load_pin=off  rst=on  en=on  explicit_period=off  period=1  dbl_ovrd=off  use_behavioral_HDL=off  use_rpm=on  implementation=Fabric  block_type=counter
      settings: xl_area=[4 7 0 7 0 0 0]  block_version=VER_STRING_GOES_HERE
  done{1..7} (7 alike) : xbsIndex_r4/Gateway Out  hdl_port=off  timing_constraint=None  locs_specified=off  LOCs={}  block_type=gatewayout
      settings: infoedit="Gateway out block.  Converts Xilinx fixed point inputs into ouputs of type Simulink integer, double, or fixed point.<P><P>Hardware notes:  In hardware these blocks become top level output ports or are discarded, depending on how they are configured."  xl_area=[0 0 0 0 1 0 0]  block_version=VER_STRING_GOES_HERE
  negedge : Subsystem
  AntA_I : Outport
  AntA_Q : Outport  Port=2
  AntB_I : Outport  Port=3
  AntB_Q : Outport  Port=4
  Vout : Outport  Port=5
  Pkt Done : Outport  Port=6
  wires:
  Q/1 -> Convert1/1
  I/1 -> Convert2/1
  Convert2/1 -> RAMs/8(I), done4/1
  Convert1/1 -> RAMs/9(Q), done3/1
  Xk_index/1 -> Addr Gen/4(Xk Index)
  [LocalReset] -> Symbol Counter/1
  Logical/1 -> RAMs/1(A We), done6/1
  Logical1/1 -> RAMs/2(B We), done5/1
  RAMs/1(AntA_I) -> AntA_I/1
  RAMs/2(AntA_Q) -> AntA_Q/1
  RAMs/3(AntB_I) -> AntB_I/1
  RAMs/4(AntB_Q) -> AntB_Q/1
  Mem Control/2(weA) -> Logical/1
  Mem Control/3(weB) -> Logical1/2
  [LocalReset] -> Addr Gen/5(rst)
  Mem Control/5(BaseRateSym) -> Addr Gen/2(BaseRateSym)
  Symbol Counter/1 -> Mem Control/1(FFTsymNum)
  Addr Gen/2(OE#A) -> RAMs/3(A OE#)
  Addr Gen/3(OE#B) -> RAMs/4(B OE#)
  Addr Gen/4(Wr Addr) -> RAMs/5(Wr Addr), done2/1
  Addr Gen/5(Rd Addr) -> RAMs/6(Rd Addr), done1/1
  IFFT Vout/1 -> Logical/2, Logical1/1, negedge/1(D)
  negedge/1(Q) -> Symbol Counter/2, Addr Gen/3(SymWrDone)
  Mem Control/4(TrainingSym) -> Addr Gen/1(TrainingSym)
  Logical2/1 -> Logical4/1
  [TxReset] -> Logical2/2
  Addr Gen/1(LastSamp) -> Logical3/2, 1 Shift Reg/3(en)
  Logical3/1 -> Logical5/1, Delay/1
  Constant2/1 -> Logical2/1
  RAMs/5(Rd Vout) -> Vout/1
  Addr Gen/6(Rd Vout) -> RAMs/7(Rd Vin), done7/1
  Logical4/1 -> [LocalReset]
  Mem Control/1(LastSym) -> 1 Shift Reg/1(d)
  [LocalReset] -> 1 Shift Reg/2(rst)
  1 Shift Reg/1(Q) -> Logical3/1
  done7/1 -> Output Buffer Scope/7
  done1/1 -> Output Buffer Scope/6
  done2/1 -> Output Buffer Scope/5
  done3/1 -> Output Buffer Scope/4
  done4/1 -> Output Buffer Scope/3
  done5/1 -> Output Buffer Scope/2
  done6/1 -> Output Buffer Scope/1
  Delay/1 -> Logical5/2
  Logical5/1 -> Pkt Done/1, Logical4/2

== system ofdm_txrx_mimo_2x/OFDM Tx MIMO/OutputMuxes ==
  note: This block adds the preamble onto the signal
      | according to what the preamble generator block sends it.
  note: Cheap output-enable circuit. Output registers are
      | brought out of reset on the first 0->1 transition in the
      | LSB of Pream Index. In other words, the outputs are
      | enabled when the preamble starts, and disabled when
      | the entire packet is finished.
  note: If CP length is 16, we don't need delay here.
      | But if we have CP less than 16, cause RAMS reading address happens later,
      | so does the data. Then data and Sel are pushed further away from preamble.
  Pream Index : Inport
  AntA I : Inport  Port=2
  AntA Q : Inport  Port=3
  AntB I : Inport  Port=4
  AntB Q : Inport  Port=5
  vin : Inport  Port=6
  Pkt Done : Inport  Port=7
  Antenna Output Scope : Scope  NumInputPorts=8
      settings: TimeRange=3000  YMin=-1~-1~0~-0.2~-0.4~-1~0~-5  YMax=1~1~1~0.6~0.4~1~400~5
  Constant1 : xbsIndex_r4/Constant  arith_type=Boolean  const=0  n_bits=1  bin_pt=0  explicit_period=off  period=2  equ=P=C  opselect=C  inp2=PCIN>>17  opr=+  inp1=P  carry=CIN  dbl_ovrd=off  block_type=constant
      settings: block_version=VER_STRING_GOES_HERE
  Constant7 : xbsIndex_r4/Constant  arith_type=Boolean  const=1  n_bits=1  bin_pt=0  explicit_period=off  period=2  equ=P=C  opselect=C  inp2=PCIN>>17  opr=+  inp1=P  carry=CIN  dbl_ovrd=off  block_type=constant
      settings: block_version=VER_STRING_GOES_HERE
  Convert : xbsIndex_r4/Convert  arith_type=Unsigned  n_bits=1  bin_pt=0  quantization=Truncate  overflow=Wrap  latency=0  dbl_ovrd=off  pipeline=off  block_type=convert
      settings: xl_area=[0, 0, 0, 0, 0, 0, 0]  block_version=VER_STRING_GOES_HERE
  Delay1 : xbsIndex_r4/Delay  en=off  latency=1  dbl_ovrd=off  reg_retiming=off  block_type=delay
      settings: infoedit=Hardware notes: A delay line is a chain, each link of which is an SRL16 followed by a flip-flop. If register retiming is enabled, the delay line is a chain of flip-flops.  block_version=VER_STRING_GOES_HERE
  Down Sample1 : xbsIndex_r4/Down Sample  sample_ratio=2  sample_phase=First Value of Frame  en=off  latency=0  dbl_ovrd=off  block_type=dsamp
      settings: block_version=VER_STRING_GOES_HERE
  Inverter : xbsIndex_r4/Inverter  en=off  latency=0  dbl_ovrd=off  block_type=inv
      settings: xl_area=[0, 0, 0, 0, 0, 0, 0]  block_version=VER_STRING_GOES_HERE
  LSB : xbsIndex_r4/Slice  nbits=1  boolean_output=on  mode=Lower Bit Location + Width  bit1=0  base1=MSB of Input  bit0=0  base0=LSB of Input  dbl_ovrd=off  block_type=slice
      settings: infoedit="Extracts a given range of bits from each input sample and presents it at the output.  The output type is ordinarily unsigned with binary point at zero, but can be Boolean when the slice is one bit wide.<P><P>Hardware notes: In hardware this block costs nothing."  block_version=VER_STRING_GOES_HERE
  Logical1 : xbsIndex_r4/Logical  logical_function=OR  inputs=2  en=off  latency=0  precision=Full  arith_type=Unsigned  n_bits=8  bin_pt=2  align_bp=on  dbl_ovrd=off  block_type=logical
      settings: xl_area=[1 0 0 1 0 0 0]  block_version=VER_STRING_GOES_HERE
  Logical3 : xbsIndex_r4/Logical  logical_function=OR  inputs=2  en=off  latency=0  precision=Full  arith_type=Unsigned  n_bits=8  bin_pt=2  align_bp=on  dbl_ovrd=off  block_type=logical
      settings: xl_area=[1 0 0 1 0 0 0]  block_version=VER_STRING_GOES_HERE
  Mux{,1..3} (4 alike) : xbsIndex_r4/Mux  inputs=2  en=off  latency=0  precision=User Defined  arith_type="Signed  (2's comp)"  n_bits=14  bin_pt=13  quantization=Truncate  overflow=Wrap  dbl_ovrd=off  block_type=mux
      settings: xl_area=[7 0 0 14 0 0 0]  block_version=VER_STRING_GOES_HERE
  Payload Running : xbsIndex_r4/Register  init=0  rst=on  en=on  dbl_ovrd=off  block_type=register
      settings: xl_area=[1 1 0 0 0 0 0]  block_version=VER_STRING_GOES_HERE
  Payload Running1 : xbsIndex_r4/Register  init=1  rst=on  en=on  dbl_ovrd=off  block_type=register
      settings: xl_area=[1 1 0 0 0 0 0]  block_version=VER_STRING_GOES_HERE
  Preamble Generation : Subsystem
  Register{,1..3} (4 alike) : xbsIndex_r4/Register  init=0  rst=on  en=off  dbl_ovrd=off  block_type=register
      settings: xl_area=[0, 0, 0, 0, 0, 0, 0]  block_version=VER_STRING_GOES_HERE
  Tx_debug_pktRunning : xbsIndex_r4/Gateway Out  hdl_port=on  timing_constraint=None  locs_specified=off  LOCs={}  block_type=gatewayout
      settings: infoedit="Gateway out block.  Converts Xilinx fixed point inputs into ouputs of type Simulink integer, double, or fixed point.<P><P>Hardware notes:  In hardware these blocks become top level output ports or are discarded, depending on how they are configured."  xl_area=[0, 0, 0, 0, 0, 0, 0]  sginterface={'Xilinx'=>{'jtaghwcosim'=>{'non_memory_mapped_port'=>'debug_pktRunning'}}}  block_version=VER_STRING_GOES_HERE
  Up Sample : xbsIndex_r4/Up Sample  sample_ratio=4  copy_samples=on  dbl_ovrd=off  block_type=usamp
      settings: infoedit="Up samples input data.  Inserted values  can be zeros or copies of the most recent input sample.<P><P>Hardware notes: No hardware is needed if inserted values are copies of the input sample; otherwise, a mux and single bit flip-flop are used."  block_version=8.2.02
  " AntA I" : Outport
  " AntA Q" : Outport  Port=2
  " AntB I" : Outport  Port=3
  " AntB Q" : Outport  Port=4
  wires:
  Logical1/1 -> Mux/1, Mux1/1, Mux2/1, Mux3/1, Antenna Output Scope/6
  Constant7/1 -> Payload Running/1
  [TxReset] -> Logical3/1
  Logical3/1 -> Down Sample1/1
  Pkt Done/1 -> Logical3/2
  vin/1 -> Payload Running/3, Logical1/2
  AntA I/1 -> Mux/3, Antenna Output Scope/7
  Mux/1 -> Register/1
  Mux1/1 -> Register1/1
  AntA Q/1 -> Mux1/3, Antenna Output Scope/8
  Constant1/1 -> Payload Running1/1
  Payload Running1/1 -> Register/2, Register1/2, Register2/2, Register3/2, Antenna Output Scope/3, Inverter/1
  LSB/1 -> Payload Running1/3
  Pream Index/1 -> LSB/1, Preamble Generation/1(Addr)
  Preamble Generation/1(AntA_I) -> Mux/2, Antenna Output Scope/4
  Preamble Generation/2(AntA_Q) -> Mux1/2, Antenna Output Scope/5
  Register/1 -> " AntA I"/1, Antenna Output Scope/1
  Register1/1 -> " AntA Q"/1, Antenna Output Scope/2
  Mux2/1 -> Register2/1
  Mux3/1 -> Register3/1
  Register2/1 -> " AntB I"/1
  Register3/1 -> " AntB Q"/1
  AntB I/1 -> Mux2/3
  AntB Q/1 -> Mux3/3
  Preamble Generation/3(AntB_I) -> Mux2/2
  Preamble Generation/4(AntB_Q) -> Mux3/2
  Down Sample1/1 -> Payload Running/2, Delay1/1
  Payload Running/1 -> Logical1/1
  Delay1/1 -> Payload Running1/2
  Inverter/1 -> Convert/1
  Convert/1 -> Up Sample/1
  Up Sample/1 -> Tx_debug_pktRunning/1
